simulink model "ddc_dev_xilfft"  (R2016b) (continued)
== system ddc_dev_xilfft == (continued)
      mask prompts: io_dir "I/O direction"; io_delay "I/O delay"; sample_period "Sample period"; names "Bitfield names [msb...lsb]"; bitwidths "Bitfield widths"; bin_pts "Bitfield binary pts"; arith_types "Bitfield types, ufix=0, fix=1, bool=2"; sim_port "Provide sim input/output?"; show_format "Print format string?"
      mask help: A 32-bit software-accessible register. Can be divided into bitfields of varying widths and types using the fields in the block mask.
      mask init:
      | swreg_init(gcb)
      settings: AttributesFormatString=1input:uf16.15 = 16 bits
      mask TypeOptions io_dir: To Processor | From Processor
      mask Callback io_dir:
      | mask_enables = get_param(gcb, 'MaskEnables');
      | mask_names = get_param(gcb, 'MaskNames');
      | if strcmp(get_param(gcb, 'io_dir'), 'To Processor'),
      |     mask_enables{ismember(mask_names, 'sample_period')} = 'off';
      | else
      |     mask_enables{ismember(mask_names, 'sample_period')} = 'on';
      | end
      | set_param(gcb, 'MaskEnables', mask_enables);
  scale_p0_ddc1 : Subsystem  Tag=xps:sw_reg  AncestorBlock=xps_library/software_register  mask(swreg){io_dir=From Processor, io_delay=1, sample_period=1, names=scale, bitwidths=16, bin_pts=15, arith_types=0, sim_port=on, show_format=on}
      mask prompts: io_dir "I/O direction"; io_delay "I/O delay"; sample_period "Sample period"; names "Bitfield names [msb...lsb]"; bitwidths "Bitfield widths"; bin_pts "Bitfield binary pts"; arith_types "Bitfield types, ufix=0, fix=1, bool=2"; sim_port "Provide sim input/output?"; show_format "Print format string?"
      mask help: A 32-bit software-accessible register. Can be divided into bitfields of varying widths and types using the fields in the block mask.
      mask init:
      | swreg_init(gcb)
      settings: AttributesFormatString=1input:uf16.15 = 16 bits
      mask TypeOptions io_dir: To Processor | From Processor
      mask Callback io_dir:
      | mask_enables = get_param(gcb, 'MaskEnables');
      | mask_names = get_param(gcb, 'MaskNames');
      | if strcmp(get_param(gcb, 'io_dir'), 'To Processor'),
      |     mask_enables{ismember(mask_names, 'sample_period')} = 'off';
      | else
      |     mask_enables{ismember(mask_names, 'sample_period')} = 'on';
      | end
      | set_param(gcb, 'MaskEnables', mask_enables);
  scale_p0_ddc2 : Subsystem  Tag=xps:sw_reg  AncestorBlock=xps_library/software_register  mask(swreg){io_dir=From Processor, io_delay=1, sample_period=1, names=scale, bitwidths=16, bin_pts=15, arith_types=0, sim_port=on, show_format=on}
      mask prompts: io_dir "I/O direction"; io_delay "I/O delay"; sample_period "Sample period"; names "Bitfield names [msb...lsb]"; bitwidths "Bitfield widths"; bin_pts "Bitfield binary pts"; arith_types "Bitfield types, ufix=0, fix=1, bool=2"; sim_port "Provide sim input/output?"; show_format "Print format string?"
      mask help: A 32-bit software-accessible register. Can be divided into bitfields of varying widths and types using the fields in the block mask.
      mask init:
      | swreg_init(gcb)
      settings: AttributesFormatString=1input:uf16.15 = 16 bits
      mask TypeOptions io_dir: To Processor | From Processor
      mask Callback io_dir:
      | mask_enables = get_param(gcb, 'MaskEnables');
      | mask_names = get_param(gcb, 'MaskNames');
      | if strcmp(get_param(gcb, 'io_dir'), 'To Processor'),
      |     mask_enables{ismember(mask_names, 'sample_period')} = 'off';
      | else
      |     mask_enables{ismember(mask_names, 'sample_period')} = 'on';
      | end
      | set_param(gcb, 'MaskEnables', mask_enables);
  scale_p0_ddc3 : Subsystem  Tag=xps:sw_reg  AncestorBlock=xps_library/software_register  mask(swreg){io_dir=From Processor, io_delay=1, sample_period=1, names=scale, bitwidths=16, bin_pts=15, arith_types=0, sim_port=on, show_format=on}
      mask prompts: io_dir "I/O direction"; io_delay "I/O delay"; sample_period "Sample period"; names "Bitfield names [msb...lsb]"; bitwidths "Bitfield widths"; bin_pts "Bitfield binary pts"; arith_types "Bitfield types, ufix=0, fix=1, bool=2"; sim_port "Provide sim input/output?"; show_format "Print format string?"
      mask help: A 32-bit software-accessible register. Can be divided into bitfields of varying widths and types using the fields in the block mask.
      mask init:
      | swreg_init(gcb)
      settings: AttributesFormatString=1input:uf16.15 = 16 bits
      mask TypeOptions io_dir: To Processor | From Processor
      mask Callback io_dir:
      | mask_enables = get_param(gcb, 'MaskEnables');
      | mask_names = get_param(gcb, 'MaskNames');
      | if strcmp(get_param(gcb, 'io_dir'), 'To Processor'),
      |     mask_enables{ismember(mask_names, 'sample_period')} = 'off';
      | else
      |     mask_enables{ismember(mask_names, 'sample_period')} = 'on';
      | end
      | set_param(gcb, 'MaskEnables', mask_enables);
  scale_p0_ddc4 : Subsystem  Tag=xps:sw_reg  AncestorBlock=xps_library/software_register  mask(swreg){io_dir=From Processor, io_delay=1, sample_period=1, names=scale, bitwidths=16, bin_pts=15, arith_types=0, sim_port=on, show_format=on}
      mask prompts: io_dir "I/O direction"; io_delay "I/O delay"; sample_period "Sample period"; names "Bitfield names [msb...lsb]"; bitwidths "Bitfield widths"; bin_pts "Bitfield binary pts"; arith_types "Bitfield types, ufix=0, fix=1, bool=2"; sim_port "Provide sim input/output?"; show_format "Print format string?"
      mask help: A 32-bit software-accessible register. Can be divided into bitfields of varying widths and types using the fields in the block mask.
      mask init:
      | swreg_init(gcb)
      settings: AttributesFormatString=1input:uf16.15 = 16 bits
      mask TypeOptions io_dir: To Processor | From Processor
      mask Callback io_dir:
      | mask_enables = get_param(gcb, 'MaskEnables');
      | mask_names = get_param(gcb, 'MaskNames');
      | if strcmp(get_param(gcb, 'io_dir'), 'To Processor'),
      |     mask_enables{ismember(mask_names, 'sample_period')} = 'off';
      | else
      |     mask_enables{ismember(mask_names, 'sample_period')} = 'on';
      | end
      | set_param(gcb, 'MaskEnables', mask_enables);
  scale_p1_ddc0 : Subsystem  Tag=xps:sw_reg  AncestorBlock=xps_library/software_register  mask(swreg){io_dir=From Processor, io_delay=1, sample_period=1, names=scale, bitwidths=16, bin_pts=15, arith_types=0, sim_port=on, show_format=on}
      mask prompts: io_dir "I/O direction"; io_delay "I/O delay"; sample_period "Sample period"; names "Bitfield names [msb...lsb]"; bitwidths "Bitfield widths"; bin_pts "Bitfield binary pts"; arith_types "Bitfield types, ufix=0, fix=1, bool=2"; sim_port "Provide sim input/output?"; show_format "Print format string?"
      mask help: A 32-bit software-accessible register. Can be divided into bitfields of varying widths and types using the fields in the block mask.
      mask init:
      | swreg_init(gcb)
      settings: AttributesFormatString=1input:uf16.15 = 16 bits
      mask TypeOptions io_dir: To Processor | From Processor
      mask Callback io_dir:
      | mask_enables = get_param(gcb, 'MaskEnables');
      | mask_names = get_param(gcb, 'MaskNames');
      | if strcmp(get_param(gcb, 'io_dir'), 'To Processor'),
      |     mask_enables{ismember(mask_names, 'sample_period')} = 'off';
      | else
      |     mask_enables{ismember(mask_names, 'sample_period')} = 'on';
      | end
      | set_param(gcb, 'MaskEnables', mask_enables);
  scale_p1_ddc1 : Subsystem  Tag=xps:sw_reg  AncestorBlock=xps_library/software_register  mask(swreg){io_dir=From Processor, io_delay=1, sample_period=1, names=scale, bitwidths=16, bin_pts=15, arith_types=0, sim_port=on, show_format=on}
      mask prompts: io_dir "I/O direction"; io_delay "I/O delay"; sample_period "Sample period"; names "Bitfield names [msb...lsb]"; bitwidths "Bitfield widths"; bin_pts "Bitfield binary pts"; arith_types "Bitfield types, ufix=0, fix=1, bool=2"; sim_port "Provide sim input/output?"; show_format "Print format string?"
      mask help: A 32-bit software-accessible register. Can be divided into bitfields of varying widths and types using the fields in the block mask.
      mask init:
      | swreg_init(gcb)
      settings: AttributesFormatString=1input:uf16.15 = 16 bits
      mask TypeOptions io_dir: To Processor | From Processor
      mask Callback io_dir:
      | mask_enables = get_param(gcb, 'MaskEnables');
      | mask_names = get_param(gcb, 'MaskNames');
      | if strcmp(get_param(gcb, 'io_dir'), 'To Processor'),
      |     mask_enables{ismember(mask_names, 'sample_period')} = 'off';
      | else
      |     mask_enables{ismember(mask_names, 'sample_period')} = 'on';
      | end
      | set_param(gcb, 'MaskEnables', mask_enables);
  scale_p1_ddc2 : Subsystem  Tag=xps:sw_reg  AncestorBlock=xps_library/software_register  mask(swreg){io_dir=From Processor, io_delay=1, sample_period=1, names=scale, bitwidths=16, bin_pts=15, arith_types=0, sim_port=on, show_format=on}
      mask prompts: io_dir "I/O direction"; io_delay "I/O delay"; sample_period "Sample period"; names "Bitfield names [msb...lsb]"; bitwidths "Bitfield widths"; bin_pts "Bitfield binary pts"; arith_types "Bitfield types, ufix=0, fix=1, bool=2"; sim_port "Provide sim input/output?"; show_format "Print format string?"
      mask help: A 32-bit software-accessible register. Can be divided into bitfields of varying widths and types using the fields in the block mask.
      mask init:
      | swreg_init(gcb)
      settings: AttributesFormatString=1input:uf16.15 = 16 bits
      mask TypeOptions io_dir: To Processor | From Processor
      mask Callback io_dir:
      | mask_enables = get_param(gcb, 'MaskEnables');
      | mask_names = get_param(gcb, 'MaskNames');
      | if strcmp(get_param(gcb, 'io_dir'), 'To Processor'),
      |     mask_enables{ismember(mask_names, 'sample_period')} = 'off';
      | else
      |     mask_enables{ismember(mask_names, 'sample_period')} = 'on';
      | end
      | set_param(gcb, 'MaskEnables', mask_enables);
  scale_p1_ddc3 : Subsystem  Tag=xps:sw_reg  AncestorBlock=xps_library/software_register  mask(swreg){io_dir=From Processor, io_delay=1, sample_period=1, names=scale, bitwidths=16, bin_pts=15, arith_types=0, sim_port=on, show_format=on}
      mask prompts: io_dir "I/O direction"; io_delay "I/O delay"; sample_period "Sample period"; names "Bitfield names [msb...lsb]"; bitwidths "Bitfield widths"; bin_pts "Bitfield binary pts"; arith_types "Bitfield types, ufix=0, fix=1, bool=2"; sim_port "Provide sim input/output?"; show_format "Print format string?"
      mask help: A 32-bit software-accessible register. Can be divided into bitfields of varying widths and types using the fields in the block mask.
      mask init:
      | swreg_init(gcb)
      settings: AttributesFormatString=1input:uf16.15 = 16 bits
      mask TypeOptions io_dir: To Processor | From Processor
      mask Callback io_dir:
      | mask_enables = get_param(gcb, 'MaskEnables');
      | mask_names = get_param(gcb, 'MaskNames');
      | if strcmp(get_param(gcb, 'io_dir'), 'To Processor'),
      |     mask_enables{ismember(mask_names, 'sample_period')} = 'off';
      | else
      |     mask_enables{ismember(mask_names, 'sample_period')} = 'on';
      | end
      | set_param(gcb, 'MaskEnables', mask_enables);
  scale_p1_ddc4 : Subsystem  Tag=xps:sw_reg  AncestorBlock=xps_library/software_register  mask(swreg){io_dir=From Processor, io_delay=1, sample_period=1, names=scale, bitwidths=16, bin_pts=15, arith_types=0, sim_port=on, show_format=on}
      mask prompts: io_dir "I/O direction"; io_delay "I/O delay"; sample_period "Sample period"; names "Bitfield names [msb...lsb]"; bitwidths "Bitfield widths"; bin_pts "Bitfield binary pts"; arith_types "Bitfield types, ufix=0, fix=1, bool=2"; sim_port "Provide sim input/output?"; show_format "Print format string?"
      mask help: A 32-bit software-accessible register. Can be divided into bitfields of varying widths and types using the fields in the block mask.
      mask init:
      | swreg_init(gcb)
      settings: AttributesFormatString=1input:uf16.15 = 16 bits
      mask TypeOptions io_dir: To Processor | From Processor
      mask Callback io_dir:
      | mask_enables = get_param(gcb, 'MaskEnables');
      | mask_names = get_param(gcb, 'MaskNames');
      | if strcmp(get_param(gcb, 'io_dir'), 'To Processor'),
      |     mask_enables{ismember(mask_names, 'sample_period')} = 'off';
      | else
      |     mask_enables{ismember(mask_names, 'sample_period')} = 'on';
      | end
      | set_param(gcb, 'MaskEnables', mask_enables);
  time_gen : Subsystem  mask{n_bits_period=fft_stages-1-2, n_bits_increment=0, n_bits_counter=48-fft_stages}
      mask prompts: n_bits_period "Period (2^?)"; n_bits_increment "Increment (2^?)"; n_bits_counter "Counter size (2^?)"
  tl_cd0_control : Subsystem  Tag=xps:sw_reg  AncestorBlock=xps_library/software_register  mask(swreg){io_dir=From Processor, io_delay=0, sample_period=1, names=load_time_lsw, bitwidths=32, bin_pts=0, arith_types=0, sim_port=on, show_format=on}
      mask prompts: io_dir "I/O direction"; io_delay "I/O delay"; sample_period "Sample period"; names "Bitfield names [msb...lsb]"; bitwidths "Bitfield widths"; bin_pts "Bitfield binary pts"; arith_types "Bitfield types, ufix=0, fix=1, bool=2"; sim_port "Provide sim input/output?"; show_format "Print format string?"
      mask help: A 32-bit software-accessible register. Can be divided into bitfields of varying widths and types using the fields in the block mask.
      mask init:
      | swreg_init(gcb)
      settings: AttributesFormatString=1input:uf32.0 = 32 bits
      mask TypeOptions io_dir: To Processor | From Processor
      mask Callback io_dir:
      | mask_enables = get_param(gcb, 'MaskEnables');
      | mask_names = get_param(gcb, 'MaskNames');
      | if strcmp(get_param(gcb, 'io_dir'), 'To Processor'),
      |     mask_enables{ismember(mask_names, 'sample_period')} = 'off';
      | else
      |     mask_enables{ismember(mask_names, 'sample_period')} = 'on';
      | end
      | set_param(gcb, 'MaskEnables', mask_enables);
  tl_cd0_control0 : Subsystem  Tag=xps:sw_reg  AncestorBlock=xps_library/software_register  mask(swreg){io_dir=From Processor, io_delay=0, sample_period=1, names=[arm load_immediate load_time_msw], bitwidths=[1 1 16], bin_pts=[0 0 0], arith_types=[2 2 0], sim_port=on, show_format=on}
      mask prompts: io_dir "I/O direction"; io_delay "I/O delay"; sample_period "Sample period"; names "Bitfield names [msb...lsb]"; bitwidths "Bitfield widths"; bin_pts "Bitfield binary pts"; arith_types "Bitfield types, ufix=0, fix=1, bool=2"; sim_port "Provide sim input/output?"; show_format "Print format string?"
      mask help: A 32-bit software-accessible register. Can be divided into bitfields of varying widths and types using the fields in the block mask.
      mask init:
      | swreg_init(gcb)
      settings: AttributesFormatString=3 inputs:b,b,uf16.0 = 18 bits
      mask TypeOptions io_dir: To Processor | From Processor
      mask Callback io_dir:
      | mask_enables = get_param(gcb, 'MaskEnables');
      | mask_names = get_param(gcb, 'MaskNames');
      | if strcmp(get_param(gcb, 'io_dir'), 'To Processor'),
      |     mask_enables{ismember(mask_names, 'sample_period')} = 'off';
      | else
      |     mask_enables{ismember(mask_names, 'sample_period')} = 'on';
      | end
      | set_param(gcb, 'MaskEnables', mask_enables);
  tl_cd1_control : Subsystem  Tag=xps:sw_reg  AncestorBlock=xps_library/software_register  mask(swreg){io_dir=From Processor, io_delay=0, sample_period=1, names=load_time_lsw, bitwidths=32, bin_pts=0, arith_types=0, sim_port=on, show_format=on}
      mask prompts: io_dir "I/O direction"; io_delay "I/O delay"; sample_period "Sample period"; names "Bitfield names [msb...lsb]"; bitwidths "Bitfield widths"; bin_pts "Bitfield binary pts"; arith_types "Bitfield types, ufix=0, fix=1, bool=2"; sim_port "Provide sim input/output?"; show_format "Print format string?"
      mask help: A 32-bit software-accessible register. Can be divided into bitfields of varying widths and types using the fields in the block mask.
      mask init:
      | swreg_init(gcb)
      settings: AttributesFormatString=1input:uf32.0 = 32 bits
      mask TypeOptions io_dir: To Processor | From Processor
      mask Callback io_dir:
      | mask_enables = get_param(gcb, 'MaskEnables');
      | mask_names = get_param(gcb, 'MaskNames');
      | if strcmp(get_param(gcb, 'io_dir'), 'To Processor'),
      |     mask_enables{ismember(mask_names, 'sample_period')} = 'off';
      | else
      |     mask_enables{ismember(mask_names, 'sample_period')} = 'on';
      | end
      | set_param(gcb, 'MaskEnables', mask_enables);
  tl_cd1_control0 : Subsystem  Tag=xps:sw_reg  AncestorBlock=xps_library/software_register  mask(swreg){io_dir=From Processor, io_delay=0, sample_period=1, names=[arm load_immediate load_time_msw], bitwidths=[1 1 16], bin_pts=[0 0 0], arith_types=[2 2 0], sim_port=on, show_format=on}
      mask prompts: io_dir "I/O direction"; io_delay "I/O delay"; sample_period "Sample period"; names "Bitfield names [msb...lsb]"; bitwidths "Bitfield widths"; bin_pts "Bitfield binary pts"; arith_types "Bitfield types, ufix=0, fix=1, bool=2"; sim_port "Provide sim input/output?"; show_format "Print format string?"
      mask help: A 32-bit software-accessible register. Can be divided into bitfields of varying widths and types using the fields in the block mask.
      mask init:
      | swreg_init(gcb)
      settings: AttributesFormatString=3 inputs:b,b,uf16.0 = 18 bits
      mask TypeOptions io_dir: To Processor | From Processor
      mask Callback io_dir:
      | mask_enables = get_param(gcb, 'MaskEnables');
      | mask_names = get_param(gcb, 'MaskNames');
      | if strcmp(get_param(gcb, 'io_dir'), 'To Processor'),
      |     mask_enables{ismember(mask_names, 'sample_period')} = 'off';
      | else
      |     mask_enables{ismember(mask_names, 'sample_period')} = 'on';
      | end
      | set_param(gcb, 'MaskEnables', mask_enables);
  tl_fd0 : Subsystem  mask(timed_latch){n_bits_time=48-fft_stages, n_bits_quantization=fft_stages, n_bits_counters=4}
      mask prompts: n_bits_time "Number bits in time to compare"; n_bits_quantization "Time offset from lsb"; n_bits_counters "Bits in status counters"
      mask help:
      | Latches load_value when armed and current_time matches requested time.
      | current_time should be in ADC samples.
      mask init:
      | set_param([gcb,'/status'], 'bitwidths', mat2str([1, repmat(n_bits_counters, 1, 2)]));
      mask Help:
      | When armed, will latch new value when current time matches the time
      | specified. The input time should be in samples and mask settings allow
      | a portion of this to be compared to cater for quantization. Arm and latch
      | counts allow the current state, pending and missed latch events to be
      | determined.
  tl_fd1 : Subsystem  mask(timed_latch){n_bits_time=48-fft_stages, n_bits_quantization=fft_stages, n_bits_counters=4}
      mask prompts: n_bits_time "Number bits in time to compare"; n_bits_quantization "Time offset from lsb"; n_bits_counters "Bits in status counters"
      mask help:
      | Latches load_value when armed and current_time matches requested time.
      | current_time should be in ADC samples.
      mask init:
      | set_param([gcb,'/status'], 'bitwidths', mat2str([1, repmat(n_bits_counters, 1, 2)]));
      mask Help:
      | When armed, will latch new value when current time matches the time
      | specified. The input time should be in samples and mask settings allow
      | a portion of this to be compared to cater for quantization. Arm and latch
      | counts allow the current state, pending and missed latch events to be
      | determined.
  write_read_arbiter : Subsystem
  wires:
  osc0/1(bus_out) -> pipeline13/1(d)
  Constant64/1 -> freq_cwg0/1(sim_1)
  scale_cwg0/1(in_scale) -> pipeline48/1(d)
  freq_cwg0/1(in_frequency) -> pipeline49/1(d)
  Constant79/1 -> freq_cwg_corr/1(sim_1)
  Constant80/1 -> scale_cwg_corr/1(sim_1)
  Constant57/1 -> scale_p0_ddc0/1(sim_1)
  pipeline49/1(q) -> cwg0/1(freq)
  pipeline48/1(q) -> cwg0/2(scale)
  [mrst] -> pipeline180/1(d)
  Constant62/1 -> rpt_length_cwg0/1(sim_1)
  rpt_en_cwg0/1(in_en) -> Convert25/1
  Constant70/1 -> rpt_en_cwg0/1(sim_1)
  Convert25/1 -> pipeline181/1(d)
  cwg0/1(bus_out) -> pipeline95/1(d)
  rpt_length_cwg0/1(in_repeat_length) -> pipeline182/1(d)
  pipeline180/1(q) -> cwg0/3(rst)
  pipeline181/1(q) -> cwg0/4(repeat_en)
  pipeline182/1(q) -> cwg0/5(repeat_length)
  cwg0/3(rpt_rst) -> Terminator61/1
  freq_cwg_corr/1(in_frequency) -> pipeline2/1(d)
  scale_cwg_corr/1(in_scale) -> pipeline1/1(d)
  pipeline147/1(q) -> osc0/3(rst)
  rpt_en_cwg_corr/1(in_en) -> pipeline3/1(d)
  rpt_length_cwg_corr/1(in_repeat_length) -> pipeline12/1(d)
  Constant96/1 -> osc0/6(en)
  [mrst] -> pipeline147/1(d)
  osc0/2(dvalid) -> Terminator65/1
  Constant97/1 -> rpt_en_cwg_corr/1(sim_1)
  Constant99/1 -> rpt_length_cwg_corr/1(sim_1)
  Constant106/1 -> cwg0/6(en)
  pipeline95/1(q) -> [cwg0]
  Constant37/1 -> scale_cwg0/1(sim_1)
  pipeline13/1(q) -> [osc_cos_ddc3], [osc_cos_ddc4], [osc_cos_ddc2], [osc_cos_ddc1], [osc_cos_ddc0]
  Constant2/1 -> [mrst]
  Constant3/1 -> [wng_rst0]
  Gateway Out7/1 -> To Workspace12/1
  Gateway Out1/1 -> To Workspace3/1
  Gateway Out3/1 -> To Workspace11/1
  Gateway Out6/1 -> To Workspace10/1
  bus_expand1/1(msb_out8) -> Gateway Out1/1
  bus_expand1/2(out7) -> Gateway Out7/1
  bus_expand1/4(out5) -> Gateway Out6/1
  bus_expand1/3(out6) -> Gateway Out3/1
  bus_expand1/8(lsb_out1) -> Gateway Out10/1
  bus_expand1/7(out2) -> Gateway Out9/1
  bus_expand1/6(out3) -> Gateway Out11/1
  bus_expand1/5(out4) -> Gateway Out8/1
  Gateway Out10/1 -> To Workspace6/1
  Gateway Out9/1 -> To Workspace7/1
  Gateway Out11/1 -> To Workspace8/1
  Gateway Out8/1 -> To Workspace9/1
  Gateway Out17/1 -> To Workspace19/1
  Gateway Out12/1 -> To Workspace24/1
  Gateway Out15/1 -> To Workspace18/1
  Gateway Out16/1 -> To Workspace17/1
  bus_expand2/1(msb_out8) -> Gateway Out12/1
  bus_expand2/2(out7) -> Gateway Out17/1
  bus_expand2/4(out5) -> Gateway Out16/1
  bus_expand2/3(out6) -> Gateway Out15/1
  bus_expand2/8(lsb_out1) -> Gateway Out13/1
  bus_expand2/7(out2) -> Gateway Out19/1
  bus_expand2/6(out3) -> Gateway Out14/1
  bus_expand2/5(out4) -> Gateway Out18/1
  Gateway Out13/1 -> To Workspace1/1
  Gateway Out19/1 -> To Workspace14/1
  Gateway Out14/1 -> To Workspace15/1
  Gateway Out18/1 -> To Workspace16/1
  [osc_cos_ddc0] -> bus_expand2/1(bus_in)
  [cwg0] -> bus_expand1/1(bus_in)
  Constant1/1 -> scale_p1_ddc0/1(sim_1)
  Constant10/1 -> freq_cwg1/1(sim_1)
  scale_cwg1/1(in_scale) -> pipeline7/1(d)
  freq_cwg1/1(in_frequency) -> pipeline8/1(d)
  pipeline8/1(q) -> cwg1/1(freq)
  pipeline7/1(q) -> cwg1/2(scale)
  [mrst] -> pipeline4/1(d)
  Constant6/1 -> rpt_length_cwg1/1(sim_1)
  rpt_en_cwg1/1(in_en) -> Convert1/1
  Constant12/1 -> rpt_en_cwg1/1(sim_1)
  Convert1/1 -> pipeline5/1(d)
  rpt_length_cwg1/1(in_repeat_length) -> pipeline6/1(d)
  pipeline4/1(q) -> cwg1/3(rst)
  pipeline5/1(q) -> cwg1/4(repeat_en)
  pipeline6/1(q) -> cwg1/5(repeat_length)
  Constant4/1 -> cwg1/6(en)
  Constant5/1 -> scale_cwg1/1(sim_1)
  edge_detect1/1 -> pipeline11/1(d)
  pipeline9/1(q) -> [cwg1]
  pipeline11/1(q) -> [sync]
  cwg0/2(dvalid) -> Terminator3/1
  Constant11/1 -> Delay14/1
  Delay14/1 -> pipeline10/1(d), edge_detect1/1
  pipeline10/1(q) -> [dvalid]
  cwg1/1(bus_out) -> pipeline9/1(d)
  cwg1/3(rpt_rst) -> Terminator2/1
  cwg1/2(dvalid) -> Terminator1/1
  Gateway Out2/1 -> To Workspace2/1
  Gateway Out4/1 -> To Workspace26/1
  Gateway Out5/1 -> To Workspace32/1
  Gateway Out20/1 -> To Workspace5/1
  Gateway Out21/1 -> To Workspace33/1
  Gateway Out23/1 -> To Workspace25/1
  Gateway Out24/1 -> To Workspace55/1
  Gateway Out22/1 -> To Workspace13/1
  Gateway Out25/1 -> To Workspace58/1
  Gateway Out57/1 -> To Workspace67/1
  Gateway Out58/1 -> To Workspace60/1
  Gateway Out60/1 -> To Workspace61/1
  Gateway Out61/1 -> To Workspace93/1
  Gateway Out67/1 -> To Workspace64/1
  Gateway Out68/1 -> To Workspace94/1
  Gateway Out59/1 -> To Workspace92/1
  [pfb0_sync] -> write_read_arbiter/1(sync)
  write_read_arbiter/1(wr_addr) -> [wr_addr]
  Gateway Out69/1 -> To Workspace4/1
  Gateway Out83/1 -> To Workspace78/1
  Gateway Out84/1 -> To Workspace83/1
  Gateway Out77/1 -> To Workspace31/1
  Gateway Out78/1 -> To Workspace84/1
  Gateway Out80/1 -> To Workspace68/1
  Gateway Out81/1 -> To Workspace85/1
  Gateway Out79/1 -> To Workspace59/1
  Gateway Out82/1 -> To Workspace69/1
  Gateway Out85/1 -> To Workspace86/1
  Gateway Out86/1 -> To Workspace79/1
  Gateway Out88/1 -> To Workspace80/1
  Gateway Out89/1 -> To Workspace89/1
  Gateway Out90/1 -> To Workspace81/1
  Gateway Out91/1 -> To Workspace90/1
  Gateway Out87/1 -> To Workspace88/1
  [pfb0_d144_p0] -> bus_expand/1(bus_in)
  Gateway Out96/1 -> To Workspace96/1
  [pfb0_dv] -> Gateway Out96/1
  bus_expand/1(msb_out8) -> Gateway Out2/1
  bus_expand/2(out7) -> Gateway Out4/1
  bus_expand/3(out6) -> Gateway Out20/1
  bus_expand/4(out5) -> Gateway Out5/1
  bus_expand/5(out4) -> Gateway Out22/1
  bus_expand/6(out3) -> Gateway Out21/1
  bus_expand/7(out2) -> Gateway Out23/1
  bus_expand/8(lsb_out1) -> Gateway Out24/1
  bus_expand6/1(msb_out8) -> Gateway Out25/1
  bus_expand6/2(out7) -> Gateway Out57/1
  bus_expand6/3(out6) -> Gateway Out58/1
  bus_expand6/4(out5) -> Gateway Out59/1
  bus_expand6/5(out4) -> Gateway Out60/1
  bus_expand6/6(out3) -> Gateway Out61/1
  bus_expand6/7(out2) -> Gateway Out67/1
  bus_expand6/8(lsb_out1) -> Gateway Out68/1
  [pfb0_d144_p1] -> bus_expand6/1(bus_in)
  [pfb1_d144_p0] -> bus_expand8/1(bus_in)
  [pfb1_d144_p1] -> bus_expand13/1(bus_in)
  bus_expand8/1(msb_out8) -> Gateway Out69/1
  bus_expand8/2(out7) -> Gateway Out83/1
  bus_expand8/3(out6) -> Gateway Out77/1
  bus_expand8/4(out5) -> Gateway Out84/1
  bus_expand8/5(out4) -> Gateway Out79/1
  bus_expand8/6(out3) -> Gateway Out78/1
  bus_expand8/7(out2) -> Gateway Out80/1
  bus_expand8/8(lsb_out1) -> Gateway Out81/1
  bus_expand13/1(msb_out8) -> Gateway Out82/1
  bus_expand13/2(out7) -> Gateway Out85/1
  bus_expand13/3(out6) -> Gateway Out86/1
  bus_expand13/4(out5) -> Gateway Out87/1
  bus_expand13/5(out4) -> Gateway Out88/1
  bus_expand13/6(out3) -> Gateway Out89/1
  bus_expand13/7(out2) -> Gateway Out90/1
  bus_expand13/8(lsb_out1) -> Gateway Out91/1
  [sync_arb] -> NB0/1(sync)
  [pfb0_in_pol0] -> NB0/2(pol0)
  NB0/2(pol0_out) -> [pfb0_d144_p0]
  NB0/3(pol1_out) -> [pfb0_d144_p1]
  NB0/4(dvalid_out) -> [pfb0_dv]
  NB0/1(sync_out) -> [pfb0_sync]
  [sync] -> DDC/1(sync)
  [dvalid] -> DDC/2(dvalid)
  [cwg0] -> DDC/3(pol0_data)
  [osc_cos_ddc0] -> DDC/4(osc0)
  scale_p0_ddc0/1(in_scale) -> DDC/5(scale0)
  [cwg1] -> DDC/6(pol1_data)
  [osc_cos_ddc0] -> DDC/7(osc1)
  scale_p1_ddc0/1(in_scale) -> DDC/8(scale1)
  DDC/1(sync_out) -> [sync_ddc0]
  DDC/2(pol0_ddc) -> [pol0_ddc0]
  DDC/3(pol1_ddc) -> [pol1_ddc0]
  DDC/4(dvalid_out) -> [dvalid_ddc0]
  [wr_addr] -> NB0/6(BRAM_wr_addr)
  [rd_addr] -> NB0/7(BRAM_rd_addr)
  [pfb0_in_pol1] -> NB0/3(pol1)
  [pfb0_dvalid] -> NB0/4(dvalid)
  [ch0_rd_en] -> NB0/5(BRAM_en)
  write_read_arbiter/4(ch1_rd_en) -> [ch0_rd_en]
  write_read_arbiter/5(ch2_rd_en) -> [ch1_rd_en]
  write_read_arbiter/6(ch3_rd_en) -> [ch2_rd_en]
  write_read_arbiter/7(ch4_rd_en) -> [ch3_rd_en]
  write_read_arbiter/8(ch5_rd_en) -> [ch4_rd_en]
  write_read_arbiter/3(rd_addr) -> [rd_addr]
  write_read_arbiter/2(wr_en) -> [wr_enable]
  [wr_enable] -> NB0/8(BRAM_wr_en)
  NB0/5(arb_en) -> [arb_en]
  [wr_enable] -> NB1/8(BRAM_wr_en)
  [rd_addr] -> NB1/7(BRAM_rd_addr)
  [wr_addr] -> NB1/6(BRAM_wr_addr)
  [ch1_rd_en] -> NB1/5(BRAM_en)
  [pfb1_dvalid] -> NB1/4(dvalid)
  [sync_arb] -> NB1/1(sync)
  [pfb1_in_pol1] -> NB1/3(pol1)
  [pfb1_in_pol0] -> NB1/2(pol0)
  NB1/5(arb_en) -> Terminator/1
  NB1/3(pol1_out) -> [pfb1_d144_p1]
  NB1/2(pol0_out) -> [pfb1_d144_p0]
  NB1/1(sync_out) -> [pfb1_sync]
  NB1/4(dvalid_out) -> [pfb1_dv]
  Gateway Out26/1 -> To Workspace20/1
  [pfb1_dv] -> Gateway Out26/1
  Gateway Out27/1 -> To Workspace21/1
  [pfb0_sync] -> Gateway Out27/1
  Gateway Out28/1 -> To Workspace22/1
  [pfb1_sync] -> Gateway Out28/1
  [pfb0_dv] -> edge_detect3/1
  [pfb1_dv] -> edge_detect4/1
  [pfb2_dv] -> edge_detect5/1
  [pfb3_dv] -> edge_detect6/1
  [pfb4_dv] -> edge_detect8/1(in), edge_detect7/1
  Logical/1 -> Counter/2
  Counter/1 -> [mux_sel]
  [mux_sel] -> Mux/1
  [mux_sel] -> Mux1/1
  Gateway Out30/1 -> To Workspace28/1
  Gateway Out37/1 -> To Workspace34/1
  bus_expand3/1(msb_out8) -> Gateway Out30/1
  bus_expand3/2(out7) -> Gateway Out37/1
  bus_expand3/3(out6) -> Gateway Out31/1
  bus_expand3/4(out5) -> Gateway Out38/1
  bus_expand3/5(out4) -> Gateway Out33/1
  bus_expand3/6(out3) -> Gateway Out32/1
  bus_expand3/7(out2) -> Gateway Out34/1
  bus_expand3/8(lsb_out1) -> Gateway Out35/1
  Gateway Out31/1 -> To Workspace27/1
  Gateway Out38/1 -> To Workspace35/1
  Gateway Out33/1 -> To Workspace29/1
  Gateway Out32/1 -> To Workspace37/1
  Gateway Out34/1 -> To Workspace30/1
  Gateway Out35/1 -> To Workspace38/1
  Mux/1 -> Gateway Out42/1, [pfb_d144_p0]
  [pfb_d144_p0] -> bus_expand3/1(bus_in)
  Constant18/1 -> Mux/2
  edge_detect3/1 -> Logical/1
  edge_detect4/1 -> Logical/2
  edge_detect5/1 -> Logical/3
  edge_detect6/1 -> Logical/4
  edge_detect7/1 -> Logical/5
  Mux1/1 -> Gateway Out43/1, [pfb_d144_p1]
  DDC1/4(dvalid_out) -> [dvalid_ddc1]
  DDC1/3(pol1_ddc) -> [pol1_ddc1]
  DDC1/2(pol0_ddc) -> [pol0_ddc1]
  DDC1/1(sync_out) -> [sync_ddc1]
  [sync] -> DDC1/1(sync)
  [dvalid] -> DDC1/2(dvalid)
  [cwg0] -> DDC1/3(pol0_data)
  [osc_cos_ddc1] -> DDC1/4(osc0)
  Constant19/1 -> scale_p0_ddc1/1(sim_1)
  scale_p0_ddc1/1(in_scale) -> DDC1/5(scale0)
  Constant20/1 -> scale_p1_ddc1/1(sim_1)
  scale_p1_ddc1/1(in_scale) -> DDC1/8(scale1)
  [osc_cos_ddc1] -> DDC1/7(osc1)
  [cwg1] -> DDC1/6(pol1_data)
  pipeline1/1(q) -> osc0/2(scale)
  pipeline2/1(q) -> osc0/1(freq)
  pipeline3/1(q) -> osc0/4(repeat_en)
  pipeline12/1(q) -> osc0/5(repeat_length)
  tl_fd0/2(enable) -> phase_compensation0/5(load_value)
  tl_fd1/1(value) -> phase_compensation1/4(delay_value)
  tl_fd1/2(enable) -> phase_compensation1/5(load_value)
  tl_fd0/1(value) -> phase_compensation0/4(delay_value)
  [clr_status] -> tl_fd0/1(rst), tl_fd1/1(rst)
  phase_compensation0/1(sync_out) -> [fdfs_sync]
  phase_compensation1/1(sync_out) -> Terminator24/1
  phase_compensation0/2(dout) -> [fdfs_d144_0]
  phase_compensation1/2(dout) -> [fdfs_d144_1]
  phase_compensation0/3(dvalid) -> [fdfs_dv]
  phase_compensation1/3(dvalid) -> Terminator25/1
  bus_expand7/1(msb_out3) -> tl_fd0/5(din)
  tl_fd0/5(dvalid) -> phase_compensation0/3(en)
  tl_fd0/3(sync_out) -> phase_compensation0/1(sync_in)
  tl_fd0/4(dout) -> phase_compensation0/2(din)
  tl_fd1/4(dout) -> phase_compensation1/2(din)
  bus_expand7/2(out2) -> tl_fd1/5(din)
  Constant29/1 -> Concat2/2
  Concat2/1 -> tl_fd1/2(current_time), [pfb_time], tl_fd0/2(current_time)
  tl_fd0/7(misco) -> Gateway Out75/1
  tl_fd0/6(time) -> Terminator5/1
  tl_fd1/6(time) -> Terminator6/1
  tl_fd1/3(sync_out) -> phase_compensation1/1(sync_in)
  tl_fd1/5(dvalid) -> phase_compensation1/3(en)
  tl_fd1/7(misco) -> Terminator7/1
  Constant22/1 -> tl_fd1/7(misci)
  time_gen/1(rd_sync_out) -> tl_fd0/4(sync_in), tl_fd1/4(sync_in)
  time_gen/2(rd_dvalid) -> tl_fd1/6(en), tl_fd0/6(en)
  time_gen/3(rd_val_out) -> Concat2/1
  [sync80] -> time_gen/1(wr_sync_in)
  [valid80] -> time_gen/2(wr_en)
  [pfb_sync] -> time_gen/4(rd_sync_in)
  [pfb_dv] -> time_gen/5(rd_en)
  Concat1/1 -> time_gen/6(rd_misc_in)
  [time80] -> time_gen/3(wr_val_in)
  [tl_cd0_load_time] -> tl_fd0/10(load_time)
  [tl_cd0_arm] -> tl_fd0/8(arm)
  [tl_cd0_load_immediate] -> tl_fd0/9(load_immediate)
  [tl_cd1_arm] -> tl_fd1/8(arm)
  [tl_cd1_load_immediate] -> tl_fd1/9(load_immediate)
  [tl_cd1_load_time] -> tl_fd1/10(load_time)
  Constant23/1 -> Delay1/1
  Constant24/1 -> [valid80]
  Constant25/1 -> [time80]
  Concat22/1 -> [ld_val0]
  Concat3/1 -> [ld_val1]
  phase0/1(in_initial) -> Reinterpret2/1
  delta_delay0/1(in_delta) -> Reinterpret1/1
  phase0/2(in_delta) -> Reinterpret3/1
  Reinterpret8/1 -> Concat22/1
  Reinterpret1/1 -> Concat22/2
  Reinterpret2/1 -> Concat22/3
  Reinterpret3/1 -> Concat22/4
  phase1/1(in_initial) -> Reinterpret5/1
  delta_delay1/1(in_delta) -> Reinterpret4/1
  phase1/2(in_delta) -> Reinterpret6/1
  Reinterpret4/1 -> Concat3/2
  Reinterpret5/1 -> Concat3/3
  Reinterpret6/1 -> Concat3/4
  Reinterpret7/1 -> Concat3/1
  delay0/1(in_initial) -> Reinterpret8/1
  delay1/1(in_initial) -> Reinterpret7/1
  tl_cd0_control0/3(in_load_time_msw) -> Concat6/1
  tl_cd0_control/1(in_load_time_lsw) -> Concat6/2
  Constant42/1 -> tl_cd0_control/1(sim_1)
  Constant40/1 -> tl_cd0_control0/3(sim_3)
  Constant41/1 -> tl_cd0_control0/2(sim_2)
  Constant43/1 -> Delay12/1
  Delay12/1 -> tl_cd0_control0/1(sim_1)
  Concat6/1 -> pipeline136/1(d)
  tl_cd0_control0/2(in_load_immediate) -> pipeline135/1(d)
  tl_cd0_control0/1(in_arm) -> pipeline134/1(d)
  tl_cd1_control0/3(in_load_time_msw) -> Concat8/1
  tl_cd1_control/1(in_load_time_lsw) -> Concat8/2
  Constant47/1 -> tl_cd1_control/1(sim_1)
  Constant45/1 -> tl_cd1_control0/3(sim_3)
  Constant46/1 -> tl_cd1_control0/2(sim_2)
  Constant48/1 -> Delay13/1
  Delay13/1 -> tl_cd1_control0/1(sim_1)
  Concat8/1 -> pipeline143/1(d)
  tl_cd1_control0/2(in_load_immediate) -> pipeline138/1(d)
  tl_cd1_control0/1(in_arm) -> pipeline137/1(d)
  pipeline134/1(q) -> [tl_cd0_arm]
  pipeline135/1(q) -> [tl_cd0_load_immediate]
  pipeline136/1(q) -> [tl_cd0_load_time]
  pipeline138/1(q) -> [tl_cd1_load_immediate]
  pipeline137/1(q) -> [tl_cd1_arm]
  pipeline143/1(q) -> [tl_cd1_load_time]
  Constant26/1 -> [clr_status]
  [ld_val1] -> tl_fd1/3(load_value)
  [pfb0_sync] -> Delay/1
  Delay/1 -> [pfb_sync]
  Gateway Out36/1 -> To Workspace36/1
  Gateway Out40/1 -> To Workspace40/1
  [pfb1_dv] -> Gateway Out39/1, Logical2/2
  [pfb0_dv] -> Gateway Out29/1, Logical2/1
  Delay1/1 -> edge_detect2/1
  edge_detect2/1 -> [sync80]
  Gateway Out42/1 -> To Workspace42/1
  Gateway Out43/1 -> To Workspace43/1
  [pfb_d144_p0] -> Concat1/1
  [pfb_d144_p1] -> Concat1/2
  Logical2/1 -> [pfb_dv]
  Gateway Out41/1 -> To Workspace45/1
  Gateway Out50/1 -> To Workspace48/1
  bus_expand4/1(msb_out8) -> Gateway Out41/1
  bus_expand4/2(out7) -> Gateway Out50/1
  bus_expand4/3(out6) -> Gateway Out45/1
  bus_expand4/4(out5) -> Gateway Out51/1
  bus_expand4/5(out4) -> Gateway Out47/1
  bus_expand4/6(out3) -> Gateway Out46/1
  bus_expand4/7(out2) -> Gateway Out48/1
  bus_expand4/8(lsb_out1) -> Gateway Out49/1
  Gateway Out45/1 -> To Workspace41/1
  Gateway Out51/1 -> To Workspace49/1
  Gateway Out47/1 -> To Workspace46/1
  Gateway Out46/1 -> To Workspace50/1
  Gateway Out48/1 -> To Workspace47/1
  Gateway Out49/1 -> To Workspace51/1
  [fdfs_d144_0] -> bus_expand4/1(bus_in)
  Gateway Out52/1 -> To Workspace52/1
  [fdfs_sync] -> Gateway Out52/1
  Gateway Out53/1 -> To Workspace53/1
  [fdfs_dv] -> Gateway Out53/1
  Counter2/1 -> pipeline29/1(d)
  Constant36/1 -> eq1/2(data_in)
  Constant28/1 -> eq1/3(we)
  Delay53/1 -> quant1/1(sync_in)
  Delay34/1 -> quant1/2(din)
  eq1/1(data_out) -> quant1/4(coeffs)
  Delay50/1 -> quant1/3(en)
  Counter4/1 -> pipeline27/1(d)
  Constant34/1 -> eq0/2(data_in)
  Constant35/1 -> eq0/3(we)
  Delay51/1 -> quant0/1(sync_in)
  Delay2/1 -> quant0/2(din)
  Delay52/1 -> quant0/3(en)
  eq0/1(data_out) -> quant0/4(coeffs)
  quant0/1(sync_out) -> [q_sync]
  quant0/2(dout) -> [q_d64_0]
  quant0/3(dvalid) -> [q_dv]
  quant0/4(of) -> [q_of0]
  quant1/2(dout) -> [q_d64_1]
  quant1/1(sync_out) -> Terminator19/1
  quant1/3(dvalid) -> Terminator21/1
  quant1/4(of) -> [q_of1]
  pipeline27/1(q) -> eq0/1(addr)
  pipeline29/1(q) -> eq1/1(addr)
  [fdfs_sync] -> Delay51/1, pipeline42/1(d)
  [fdfs_d144_0] -> Delay2/1
  [fdfs_dv] -> Delay52/1, pipeline41/1(d)
  pipeline41/1(q) -> Counter4/2
  pipeline42/1(q) -> Counter4/1
  pipeline45/1(q) -> Counter2/2
  [fdfs_sync] -> Delay53/1, pipeline14/1(d)
  [fdfs_d144_1] -> Delay34/1
  [fdfs_dv] -> Delay50/1, pipeline45/1(d)
  pipeline14/1(q) -> Counter2/1
  [clr_status] -> Subsystem5/1(rst)
  Subsystem5/1(out0) -> quant_status/1(out_pol0_or_cnt)
  Relational/1 -> Subsystem5/2(in0)
  Constant27/1 -> Relational/2
  [q_of0] -> Relational/1
  Relational3/1 -> Subsystem5/3(in1)
  [q_of1] -> Relational3/1
  Constant33/1 -> Relational3/2
  Subsystem5/2(out1) -> quant_status/2(out_pol1_or_cnt)
  Gateway Out54/1 -> To Workspace56/1
  Gateway Out65/1 -> To Workspace63/1
  bus_expand5/1(msb_out8) -> Gateway Out54/1
  bus_expand5/2(out7) -> Gateway Out65/1
  bus_expand5/3(out6) -> Gateway Out55/1
  bus_expand5/4(out5) -> Gateway Out66/1
  bus_expand5/5(out4) -> Gateway Out62/1
  bus_expand5/6(out3) -> Gateway Out56/1
  bus_expand5/7(out2) -> Gateway Out63/1
  bus_expand5/8(lsb_out1) -> Gateway Out64/1
  Gateway Out55/1 -> To Workspace54/1
  Gateway Out66/1 -> To Workspace65/1
  Gateway Out62/1 -> To Workspace57/1
  Gateway Out56/1 -> To Workspace66/1
  Gateway Out63/1 -> To Workspace62/1
  Gateway Out64/1 -> To Workspace70/1
  [q_d64_0] -> bus_expand5/1(bus_in)
  Gateway Out70/1 -> To Workspace71/1
  [q_sync] -> Gateway Out70/1
  Gateway Out71/1 -> To Workspace72/1
  [q_dv] -> Gateway Out71/1
  Gateway Out44/1 -> To Workspace44/1
  [mux_sel] -> Delay3/1
  [spec_num] -> Gateway Out44/1
  [pfb_sync] -> Gateway Out40/1
  [pfb_dv] -> Gateway Out36/1
  [pfb1_d144_p1] -> Delay19/1
  [pfb0_d144_p1] -> Delay18/1
  Constant38/1 -> Mux1/2
  [wr_enable] -> NB2/8(BRAM_wr_en)
  [rd_addr] -> NB2/7(BRAM_rd_addr)
  [sync_arb] -> NB2/1(sync)
  [pfb2_in_pol0] -> NB2/2(pol0)
  [pfb2_in_pol1] -> NB2/3(pol1)
  [pfb2_dvalid] -> NB2/4(dvalid)
  [ch2_rd_en] -> NB2/5(BRAM_en)
  [wr_addr] -> NB2/6(BRAM_wr_addr)
  [wr_enable] -> NB3/8(BRAM_wr_en)
  [rd_addr] -> NB3/7(BRAM_rd_addr)
  [sync_arb] -> NB3/1(sync)
  [pfb3_in_pol0] -> NB3/2(pol0)
  [pfb3_in_pol1] -> NB3/3(pol1)
  [pfb3_dvalid] -> NB3/4(dvalid)
  [ch3_rd_en] -> NB3/5(BRAM_en)
  [wr_addr] -> NB3/6(BRAM_wr_addr)
  [wr_enable] -> NB4/8(BRAM_wr_en)
  [rd_addr] -> NB4/7(BRAM_rd_addr)
  [sync_arb] -> NB4/1(sync)
  [pfb4_in_pol0] -> NB4/2(pol0)
  [pfb4_in_pol1] -> NB4/3(pol1)
  [pfb4_dvalid] -> NB4/4(dvalid)
  [ch4_rd_en] -> NB4/5(BRAM_en)
  [wr_addr] -> NB4/6(BRAM_wr_addr)
  NB2/1(sync_out) -> [pfb2_sync]
  NB2/2(pol0_out) -> [pfb2_d144_p0]
  NB2/3(pol1_out) -> [pfb2_d144_p1]
  NB2/4(dvalid_out) -> [pfb2_dv]
  NB3/1(sync_out) -> [pfb3_sync]
  NB3/2(pol0_out) -> [pfb3_d144_p0]
  NB3/3(pol1_out) -> [pfb3_d144_p1]
  NB3/4(dvalid_out) -> [pfb3_dv]
  NB2/5(arb_en) -> Terminator8/1
  NB3/5(arb_en) -> Terminator9/1
  NB4/5(arb_en) -> Terminator10/1
  NB4/1(sync_out) -> [pfb4_sync]
  NB4/2(pol0_out) -> [pfb4_d144_p0]
  NB4/3(pol1_out) -> [pfb4_d144_p1]
  NB4/4(dvalid_out) -> [pfb4_dv]
  [pfb2_dv] -> Gateway Out72/1, Logical2/3
  [pfb3_dv] -> Gateway Out73/1, Logical2/4
  [pfb4_dv] -> Gateway Out74/1, Logical2/5
  [pfb4_d144_p1] -> Delay22/1
  [pfb3_d144_p1] -> Delay21/1
  [pfb2_d144_p1] -> Delay20/1
  Gateway Out29/1 -> To Workspace23/1
  Gateway Out39/1 -> To Workspace39/1
  Gateway Out72/1 -> To Workspace73/1
  Gateway Out73/1 -> To Workspace74/1
  Gateway Out74/1 -> To Workspace75/1
  [pfb0_sync] -> edge_detect/1
  [pfb0_d144_p0] -> Delay4/1
  [pfb1_d144_p0] -> Delay5/1
  [pfb2_d144_p0] -> Delay6/1
  [pfb3_d144_p0] -> Delay7/1
  [pfb4_d144_p0] -> Delay8/1
  write_read_arbiter/9(sync_out) -> [sync_arb]
  [pol0_ddc0] -> Delay9/1
  Delay9/1 -> [pfb0_in_pol0]
  [pol1_ddc0] -> Delay10/1
  Delay10/1 -> [pfb0_in_pol1]
  [dvalid_ddc0] -> Delay11/1
  Delay11/1 -> [pfb0_dvalid]
  [pol0_ddc1] -> Delay17/1
  Delay17/1 -> [pfb1_in_pol0]
  [pol1_ddc1] -> Delay15/1
  Delay15/1 -> [pfb1_in_pol1]
  [dvalid_ddc1] -> Delay16/1
  Delay16/1 -> [pfb1_dvalid]
  Logical1/1 -> Counter/1
  edge_detect8/1(out) -> Logical1/2
  edge_detect/1 -> Logical1/1
  Delay4/1 -> Mux/3
  Delay5/1 -> Mux/4
  Delay6/1 -> Mux/5
  Delay7/1 -> Mux/6
  Delay8/1 -> Mux/7
  Delay20/1 -> Mux1/5
  Delay22/1 -> Mux1/7
  Delay18/1 -> Mux1/3
  Delay19/1 -> Mux1/4
  Delay21/1 -> Mux1/6
  [spec_num] -> Concat1/3
  time_gen/4(rd_misc_out) -> bus_expand7/1(bus_in)
  bus_expand7/3(lsb_out1) -> [fd_spec_num], tl_fd0/7(misci)
  Gateway Out75/1 -> To Workspace76/1
  [arb_en] -> write_read_arbiter/2(enable)
  [sync] -> DDC2/1(sync)
  [dvalid] -> DDC2/2(dvalid)
  [cwg0] -> DDC2/3(pol0_data)
  [osc_cos_ddc2] -> DDC2/4(osc0)
  Constant15/1 -> scale_p0_ddc2/1(sim_1)
  scale_p0_ddc2/1(in_scale) -> DDC2/5(scale0)
  Constant16/1 -> scale_p1_ddc2/1(sim_1)
  scale_p1_ddc2/1(in_scale) -> DDC2/8(scale1)
  [osc_cos_ddc2] -> DDC2/7(osc1)
  [cwg1] -> DDC2/6(pol1_data)
  DDC2/1(sync_out) -> [sync_ddc2]
  DDC2/2(pol0_ddc) -> [pol0_ddc2]
  DDC2/3(pol1_ddc) -> [pol1_ddc2]
  DDC2/4(dvalid_out) -> [dvalid_ddc2]
  [pol0_ddc2] -> Delay25/1
  Delay25/1 -> [pfb2_in_pol0]
  [pol1_ddc2] -> Delay23/1
  Delay23/1 -> [pfb2_in_pol1]
  [dvalid_ddc2] -> Delay24/1
  Delay24/1 -> [pfb2_dvalid]
  [sync] -> DDC3/1(sync)
  [dvalid] -> DDC3/2(dvalid)
  [cwg0] -> DDC3/3(pol0_data)
  [osc_cos_ddc3] -> DDC3/4(osc0)
  Constant17/1 -> scale_p0_ddc3/1(sim_1)
  scale_p0_ddc3/1(in_scale) -> DDC3/5(scale0)
  Constant21/1 -> scale_p1_ddc3/1(sim_1)
  scale_p1_ddc3/1(in_scale) -> DDC3/8(scale1)
  [osc_cos_ddc3] -> DDC3/7(osc1)
  [cwg1] -> DDC3/6(pol1_data)
  DDC3/4(dvalid_out) -> [dvalid_ddc3]
  DDC3/3(pol1_ddc) -> [pol1_ddc3]
  DDC3/2(pol0_ddc) -> [pol0_ddc3]
  DDC3/1(sync_out) -> [sync_ddc3]
  [pol0_ddc3] -> Delay28/1
  Delay28/1 -> [pfb3_in_pol0]
  [pol1_ddc3] -> Delay26/1
  Delay26/1 -> [pfb3_in_pol1]
  [dvalid_ddc3] -> Delay27/1
  Delay27/1 -> [pfb3_dvalid]
  [sync] -> DDC4/1(sync)
  [dvalid] -> DDC4/2(dvalid)
  [cwg0] -> DDC4/3(pol0_data)
  [osc_cos_ddc4] -> DDC4/4(osc0)
  Constant30/1 -> scale_p0_ddc4/1(sim_1)
  scale_p0_ddc4/1(in_scale) -> DDC4/5(scale0)
  Constant31/1 -> scale_p1_ddc4/1(sim_1)
  scale_p1_ddc4/1(in_scale) -> DDC4/8(scale1)
  [osc_cos_ddc4] -> DDC4/7(osc1)
  [cwg1] -> DDC4/6(pol1_data)
  DDC4/1(sync_out) -> [sync_ddc4]
  DDC4/2(pol0_ddc) -> [pol0_ddc4]
  DDC4/3(pol1_ddc) -> [pol1_ddc4]
  DDC4/4(dvalid_out) -> [dvalid_ddc4]
  Delay31/1 -> [pfb4_in_pol0]
  Delay29/1 -> [pfb4_in_pol1]
  Delay30/1 -> [pfb4_dvalid]
  [pol0_ddc4] -> Delay31/1
  [pol1_ddc4] -> Delay29/1
  [dvalid_ddc4] -> Delay30/1
  Delay3/1 -> [spec_num]
  [ld_val0] -> tl_fd0/3(load_value)
